simulink model "MCVT_Integration"  (R2020a)
settings: Solver=ode8  StartTime=0.0  StopTime=T_end  FixedStep=dt  RelTol=1e-3  EnableMultiTasking=on
values from neighbouring files (symbols this model uses):
  assumed_power_voltage = 1.25e2    (= 125)    [MCVT_Input_File.m]    (also in MCVT_Run_QuickCheck.m)
  battery_power_toggle = 1    [MCVT_Input_File.m]    (also in MCVT_Run_QuickCheck.m)
  battery_trickle_charge_param = 0.1    [MCVT_Input_File.m]    (also in MCVT_Run_QuickCheck.m)
  battery_trickle_leakage_param = 0.01    [MCVT_Input_File.m]    (also in MCVT_Run_QuickCheck.m)
  damage_case_id = 3    [MCVT_Input_File.m]    (also in MCVT_Run_QuickCheck.m = 1)
  dt = 1/fs    (= 0.001)    [MCVT_Run_QuickCheck.m]    (also in MCVT_Sim_Set.m)
  dt_2 = 1/fs_2    (= 0.001)    [MCVT_Run_QuickCheck.m]    (also in MCVT_Sim_Set.m)
  dt_3 = 1/fs_3    (= 0.001)    [MCVT_Run_QuickCheck.m]    (also in MCVT_Sim_Set.m)
  dt_5 = 1/fs_5    (= 0.005)    [MCVT_Run_QuickCheck.m]    (also in MCVT_Sim_Set.m)
  dt_8 = 1/fs_8    (= 0.05)    [MCVT_Run_QuickCheck.m]    (also in MCVT_Sim_Set.m)
  dt_9 = 1/fs_9    (= 0.001)    [MCVT_Run_QuickCheck.m]    (also in MCVT_Sim_Set.m)
  dust_met_duration = 3    [MCVT_Input_File.m]    (also in MCVT_Run_QuickCheck.m = 2)
  ECLSS_indoor_set_temperature = 298.15    [MCVT_Input_File.m]    (also in MCVT_Run_QuickCheck.m)
  energy_store_max_o = 150    [MCVT_Input_File.m]    (also in MCVT_Run_QuickCheck.m)
  energy_store_o = 120    [MCVT_Input_File.m]    (also in MCVT_Run_QuickCheck.m)
  fs = 1000    [MCVT_Run_QuickCheck.m]    (also in MCVT_Sim_Set.m)
  gain_charge_param = 1    [MCVT_Input_File.m]    (also in MCVT_Run_QuickCheck.m)
  ground_temperature = 373    [MCVT_Habitat_Design_Input_File.m]    (also in MCVT_Run_QuickCheck.m)
  I_max_charge = 800    [MCVT_Input_File.m]    (also in MCVT_Run_QuickCheck.m)
  I_max_discharge = 800    [MCVT_Input_File.m]    (also in MCVT_Run_QuickCheck.m)
  initial_pressure = 1e5    (= 100000)    [MCVT_Input_File.m]    (also in MCVT_Run_QuickCheck.m)
  initial_temperature = 295    [MCVT_Input_File.m]    (also in MCVT_Run_QuickCheck.m)
  interior_leak_rate = 0.00415    [MCVT_Input_File.m]    (also in MCVT_Run_QuickCheck.m)
  launch_dust_accum_start_time = 10    [MCVT_Input_File.m]    (also in MCVT_Run_QuickCheck.m = 2)
  launch_dust_accum_stop_time = 12    [MCVT_Input_File.m]    (also in MCVT_Run_QuickCheck.m = 3)
  meteorite_impact_time = 2    [MCVT_Input_File.m]    (also in MCVT_Run_QuickCheck.m = 1)
  nuclear_power_toggle = 1    [MCVT_Input_File.m]    (also in MCVT_Run_QuickCheck.m)
  print_interval = 30    [MCVT_Input_File.m]
  SOC_lower_thres = 0.5    [MCVT_Input_File.m]    (also in MCVT_Run_QuickCheck.m)
  SOC_upper_thres = 0.9    [MCVT_Input_File.m]    (also in MCVT_Run_QuickCheck.m)
  solar_power_toggle = 1    [MCVT_Input_File.m]    (also in MCVT_Run_QuickCheck.m)
  space_temperature = 4    [MCVT_Habitat_Design_Input_File.m]    (also in MCVT_Run_QuickCheck.m)
  Start_angle = 90    [MCVT_Input_File.m]    (also in MCVT_Run_QuickCheck.m)
undefined symbols (not defined in the file or in its folder): A_flow_restrictor, ACTIVITY_LOOKUP_TABLE, air, Aref, Bmap, Bmap2, Bmap3, comms, DiscIntNeverNeededParam, DmgSPL, dust_parameters, ECLSS_dust_damage_repair_time, ECLSS_heater_capacity, ECLSS_IPS_restrictor, ECLSS_IPS_setpointPressure, ECLSS_paint_damage_repair_time, ECLSS_radiatorLoop_discreteNumber, ECLSS_Vst, emptyActivity, expected_heat_add, FI, habitat, HIEM2SMM, HIEM2STM, HIEMDamageElements, impact_numbering, initial_SPLT_damage_index, input_air, input_Cond, input_CP, input_discretization, input_EV, input_Evap, input_radiatorLoop, K_ECLSS_dust_single, K_ECLSS_paint_single, K_Structure_damage_single, Kref, kW_to_W, meteoroidDustDep, mFRF, ndelays, nominalDustDep, noverlap, nuclear_toggle_gain, output_limits, panel_paint0, Ps0, reference_temperature, RepairTime_ECLSS_factor, Sensor_Location_SMM, SMM_T0, SMM_T1, SMM_T2, SMM_T3, SMM_T4, SMM_T4_0, SMM_T4_1, SMM_T4_2, solar_FDD_K ... (+73)
libraries: neural x350, simulink x39, xpcutilitieslib x7, xpcrtudplib x7, dspstat3 x1
(part 3 of 43)

== system MCVT_Integration/Sys 6 - Health Management and Agents/ Agent System/Agent Module ==
  Intervention_Pgm : Inport
  Action Scheduler : Subsystem
  Agent Memory : Subsystem
  Bus Creator : BusCreator  Inputs=3
  Bus Creator1 : BusCreator  Inputs=3
  MATLAB Function : MATLAB Function  TreatAsAtomicUnit=on
  Plant Model : Subsystem
  Terminator : Terminator
  Unit Delay : UnitDelay  InitialCondition=false  SampleTime=-1  HasFrameUpgradeWarning=on
  programInfo : Outport
  Agent_Memory_States : Outport  Port=2
  AgentPlantStates : Outport  Port=3
  AgentComms : Outport  Port=4
  wires:
  Action Scheduler/3(currActParams) -> Plant Model/2(actionParams)
  Action Scheduler/5(actSampleTime) -> Plant Model/4(actSampleTime)
  Unit Delay/1 -> Agent Memory/1(currAcvtDone)
  Intervention_Pgm/1 -> Agent Memory/2(Intervention_Pgm)
  Action Scheduler/2(currActID) -> MATLAB Function/1(actionID), Bus Creator1/2, Plant Model/1(actionID)  "actionID"
  Action Scheduler/1(currAcvtDone) -> Unit Delay/1
  Action Scheduler/4(currActDone) -> Plant Model/3(actionDone)
  Agent Memory/1(currPgm) -> Action Scheduler/1(CURR_TODO)  "CURR_TODO"
  Plant Model/2(Comms_SubSys_Combined) -> AgentComms/1
  Agent Memory/4(currAcvtID) -> Bus Creator/3, Bus Creator1/1  "currAcvtID"
  Plant Model/1(States) -> AgentPlantStates/1
  MATLAB Function/1(cActionID) -> Bus Creator1/3  "cActionID"
  Bus Creator/1 -> Agent_Memory_States/1
  Agent Memory/3(activityIDs) -> Bus Creator/2  "Activity_IDs"
  Bus Creator1/1 -> programInfo/1
  Agent Memory/2(filledMemSlots) -> Bus Creator/1  "Filled_Memory_Slots"
  Plant Model/3(action_done_flags) -> Terminator/1

== system MCVT_Integration/Sys 6 - Health Management and Agents/ Agent System/Interact_with_Susystems ==
  note: Agent Interactions with Electro-Mechanical Subsystems
      | The `Agent_Subsys_Comms` arre packaged as follows:
      | -------------------------------------------------------------------------
      | | Index | Relevant Electro-mechanical Susystem |
      | --------------------------------------------------------------------------
      | | 1 | Nuclear Power Generation |
      | | 2 | Solar Power Generation |
      | | 3 | Structure |
      | | 4 | ECLSS |
      | --------------------------------------------------------------------------
  Agent_SubSys_Comms : Inport
  AgentCommBus : BusCreator  Inputs=4
  Selector : Selector  NumberOfDimensions=2  InputPortWidth=3  IndexOptions=Select all,Index vector (dialog)  Indices=[1 3],1  OutputSizes=1,1
  Selector1 : Selector  NumberOfDimensions=2  InputPortWidth=3  IndexOptions=Select all,Index vector (dialog)  Indices=[1 3],2  OutputSizes=1,1
  Selector2 : Selector  NumberOfDimensions=2  InputPortWidth=3  IndexOptions=Select all,Index vector (dialog)  Indices=[1 3],3  OutputSizes=1,1
  Selector3 : Selector  NumberOfDimensions=2  InputPortWidth=3  IndexOptions=Select all,Index vector (dialog)  Indices=[1 3],4  OutputSizes=1,1
  Agent_Comms : Outport
  wires:
  AgentCommBus/1 -> Agent_Comms/1
  Agent_SubSys_Comms/1 -> Selector/1, Selector1/1, Selector3/1, Selector2/1
  Selector2/1 -> AgentCommBus/3  "Repair_Comms_Structure"
  Selector3/1 -> AgentCommBus/4  "Repair_Comms_ECLSS"
  Selector/1 -> AgentCommBus/1  "Repair_Comms_NPG"
  Selector1/1 -> AgentCommBus/2  "Repair_Comms_SPG"

== system MCVT_Integration/Sys 6 - Health Management and Agents/ Agent System/Viz ==
  AgentStates : Inport
  Agent_Comms : Inport  Port=2
  Agent Interaction : Scope  NumInputPorts=4
  Agent Memory : Scope  NumInputPorts=3
  Agent States : Scope  NumInputPorts=3
  Bus Selector : BusSelector  OutputSignals=Agent_Program_Info.currAcvtID,Agent_Program_Info.cActionID,Agent_Memory_States.Filled_Memory_Slots,Agent_Plant_States
  Bus Selector1 : BusSelector  OutputSignals=Repair_Comms_NPG,Repair_Comms_SPG,Repair_Comms_Structure,Repair_Comms_ECLSS
  Selector : Selector  InputPortWidth=3  IndexOptions=Index vector (dialog)  Indices=1  OutputSizes=1
  Selector1 : Selector  InputPortWidth=3  IndexOptions=Index vector (dialog)  Indices=2  OutputSizes=1
  Selector2 : Selector  InputPortWidth=3  IndexOptions=Index vector (dialog)  Indices=3  OutputSizes=1
  wires:
  AgentStates/1 -> Bus Selector/1
  Agent_Comms/1 -> Bus Selector1/1
  Bus Selector1/1 -> Agent Interaction/1  "<Repair_Comms_NPG>"
  Bus Selector1/2 -> Agent Interaction/2  "<Repair_Comms_SPG>"
  Bus Selector1/3 -> Agent Interaction/3  "<Repair_Comms_Structure>"
  Bus Selector1/4 -> Agent Interaction/4  "<Repair_Comms_ECLSS>"
  Bus Selector/1 -> Agent Memory/1  "<currAcvtID>"
  Bus Selector/2 -> Agent Memory/2  "<cActionID>"
  Bus Selector/3 -> Agent Memory/3  "<Filled_Memory_Slots>"
  Bus Selector/4 -> Selector2/1, Selector1/1, Selector/1  "<Agent_Plant_States>"
  Selector/1 -> Agent States/1  "soc"
  Selector1/1 -> Agent States/2  "mobilityHS"
  Selector2/1 -> Agent States/3  "position"

== system MCVT_Integration/Sys 6 - Health Management and Agents/Decision Maker/Simulink Communication Modules ==
  note: Sockets for FDD - ECLSS
  note: Sockets for FDD - Power Systems
  note: Socket for FDD - Structural System
  note: Simulated / Physics-based FDD Time Signatures at simulation frequency (fs = 1000 Hz)
  note: Simulink Communication APIs
  Sub6_Cyber_Inputs : Inport
  Agent_States : Inport  Port=2
  Agent/MCVT API from HMS : Subsystem
  Agent/MCVT API to HMS : Subsystem
  Bus Selector : BusSelector  OutputSignals=Sys2_out_DamageProbabilities.Sys2_Out_FDD_Structure_Damage_xe,Sys3_Out_PowerSystemDamageInformation.SolarPV_FDD_simu,Sys3_Out_PowerSystemDamageInformation.Nuclear_Dust_FDD,Sys5_Out_Damage_Info.FDD_ECLSS_Dust_xe,Sys5_Out_Damage_Info.FDD_ECLSS_Paint_xe
  Bus Selector1 : BusSelector  OutputSignals=Agent_Memory_States.currAcvtID
  Constant : Constant  Value=0.1
  Constant1 : Constant  Value=0.1*ones(4,1)
  Constant2 : Constant  Value=0
  ECLSS_Dust/MCVT API to HMS : Subsystem
  ECLSS_Paint/MCVT API to HMS : Subsystem
  NPG_Dust/MCVT API to HMS : Subsystem
  Solar_PV/MCVT API to HMS : Subsystem
  Structure_Dmg/MCVT API to HMS : Subsystem
  HMS_Suggested_Activity : Outport
  wires:
  Sub6_Cyber_Inputs/1 -> Bus Selector/1
  Bus Selector/1 -> [FDD_Str_Dmg_xe]  "<Sys2_Out_FDD_Structure_Damage_xe>"
  Bus Selector/2 -> [FDD_SPG_Dust_xe]  "<SolarPV_FDD_simu>"
  Bus Selector/3 -> [FDD_NPG_Dust]  "<Nuclear_Dust_FDD>"
  Bus Selector/4 -> [FDD_ECLSS_Dust_xe]  "<FDD_ECLSS_Dust_xe>"
  Bus Selector/5 -> [FDD_ECLSS_Paint_xe]  "<FDD_ECLSS_Paint_xe>"
  [FDD_ECLSS_Dust_xe] -> ECLSS_Dust/MCVT API to HMS/1(ECLSS_Dust_xe)
  [FDD_ECLSS_Paint_xe] -> ECLSS_Paint/MCVT API to HMS/1(ECLSS_Paint_xe)
  Agent_States/1 -> Bus Selector1/1
  Bus Selector1/1 -> [Agent_States]  "<currAcvtID>"
  [Agent_States] -> Agent/MCVT API to HMS/1(Agent_States)
  Agent/MCVT API from HMS/1(HMS_Suggested_Activity) -> HMS_Suggested_Activity/1
  Constant/1 -> Structure_Dmg/MCVT API to HMS/1(Structure_FDD_Damage_xe)
  Constant1/1 -> Solar_PV/MCVT API to HMS/1(FDD_SPG_Dust_xe)
  Constant2/1 -> NPG_Dust/MCVT API to HMS/1(FDD_NPG_Dust)

== system MCVT_Integration/Sys 6 - Health Management and Agents/Decision Maker/Viz ==
  Sub6_Cyber_Inputs : Inport
  Sys6_Phy_Inputs : Inport  Port=2
  Bus Selector : BusSelector  OutputSignals=Sys2_out_DamageProbabilities.Sys2_Out_FDD_Structure_Damage_xe,Sys3_Out_PowerSystemDamageInformation.SolarPV_FDD_simu,Sys3_Out_PowerSystemDamageInformation.Nuclear_Dust_FDD,Sys5_Out_Damage_Info.FDD_ECLSS_Dust_xe,Sys5_Out_Damage_Info.FDD_ECLSS_Paint_xe,Sys2_out_DamageProbabilities.Sys2_Out_PanelDamageProbabilities
  Bus Selector1 : BusSelector  OutputSignals=Sys2_Total_Damage_to_Sys6,Sys3_Out_Physical_Inputs_Agents.Sub3_solarPV_DRout,Sys3_Out_Physical_Inputs_Agents.Sub3_Nuclear_Out_Accumulated_Dust_mg_cm2
  FDD Outputs : Scope  NumInputPorts=6
  Physical States : Scope  NumInputPorts=3
  wires:
  Sub6_Cyber_Inputs/1 -> Bus Selector/1
  Bus Selector/1 -> FDD Outputs/1  "<Sys2_Out_FDD_Structure_Damage_xe>"
  Bus Selector/2 -> FDD Outputs/2  "<SolarPV_FDD_simu>"
  Bus Selector/3 -> FDD Outputs/3  "<Nuclear_Dust_FDD>"
  Bus Selector/4 -> FDD Outputs/4  "<FDD_ECLSS_Dust_xe>"
  Bus Selector/5 -> FDD Outputs/5  "<FDD_ECLSS_Paint_xe>"
  Sys6_Phy_Inputs/1 -> Bus Selector1/1
  Bus Selector1/1 -> Physical States/1  "<Sys2_Total_Damage_to_Sys6>"
  Bus Selector1/2 -> Physical States/2  "<Sub3_solarPV_DRout>"
  Bus Selector1/3 -> Physical States/3  "<Sub3_Nuclear_Out_Accumulated_Dust_mg_cm2>"
  Bus Selector/6 -> FDD Outputs/6  "<Sys2_Out_PanelDamageProbabilities>"

== system MCVT_Integration/Sys 6 - Health Management and Agents/Decision Maker/module3 ==
  Sub6_Cyber_Inputs : Inport
  Bus Selector : BusSelector  OutputSignals=Sys3_Out_PowerSystemDamageInformation.Nuclear_Dust_FDD,Sys3_Out_PowerSystemDamageInformation.SolarPV_FDD,Sys2_out_DamageProbabilities.Sys2_Out_PanelDamageProbabilities
  Mux : Mux  Inputs=3
  test_inputs : Outport
  wires:
  Sub6_Cyber_Inputs/1 -> Bus Selector/1
  Bus Selector/1 -> Mux/1  "<Nuclear_Dust_FDD>"
  Bus Selector/2 -> Mux/2  "<SolarPV_FDD>"
  Bus Selector/3 -> Mux/3  "<Sys2_Out_PanelDamageProbabilities>"
  Mux/1 -> test_inputs/1

== system MCVT_Integration/Sys2 - Structural System/Sys2_Structural_System/3D Structural Protective Layer-Thermal (SPL-T) ==
  Sys2.4_STM2SPL_MemoryBock : Inport
  Sys2.4_In_Solar_Irradiation_(from_Sys9) : Inport  Port=2
  Sys2.4_In_Damage_SPL : Inport  Port=3
  Sys2.4_In_Meteorite_Impact_Location : Inport  Port=4
  Clock : Clock
  Fir_SPL : ToWorkspace  VariableName=Fir_SPL  SampleTime=-1
  Sec_SPL : ToWorkspace  VariableName=Sec_SPL  SampleTime=-1
  Start_angle : Constant  Value=Start_angle
  Structural Protective Layer-Thermal (SPL-T) : MATLABSystem  System=SPLT  emis_reg=regolith.emissivity  abs_reg=regolith.absorptivity  sigma=sb_sigma  rho_reg=regolith.density  k_reg=regolith.thermal_conductivity  c_reg=regolith.specific_heat  k_con=concrete.thermal_conductivity  Tspace=space_temperature  R=habitat.inner_radius+habitat.dome_thickness  d=habitat.spl_thickness  d_STM=habitat.dome_thickness  Trepair=repair_temperature  Tfoundation=foundation_temperature  SimulateUsing=Code generation
      mask Type: SPLT
      mask Display:
      | disp('SPLT');
      | port_label('input',1,'STM2SPL1');
      | port_label('input',2,'Sb_tot');
      | port_label('input',3,'t');
      | port_label('input',4,'dt_2');
      | port_label('input',5,'Dam_SPL');
      | port_label('input',6,'ImpLoc');
      | port_label('input',7,'Start_angle');
      | port_label('input',8,'T_SPL_initialize');
      | port_label('output',1,'SPL2STM');
      | port_label('output',2,'Index_Damage');
      | port_label('output',3,'T_SPL_Ext');
      | port_label('output',4,'T_Fir');
      | port_label('output',5,'T_Sec');
      | port_label('output',6,'T_Thi');
      | port_label('output',7,'SPL_Sec');
      | port_label('output',8,'Fir_SPL');
      | port_label('output',9,'Sec_SPL');
      | port_label('output',10,'Thi_SPL');
  T_Fir : Scope  Commented=on
  T_SPL_initialize : Constant  Value=T_SPL_initialize  VectorParams1D=off
  T_Sec : Scope  Commented=on
  T_Thi : Scope  Commented=on
  Thi_SPL : ToWorkspace  VariableName=Thi_SPL  SampleTime=-1
  Time step : Constant  Value=dt_2
  Sys2.4_SPL2STM_MemoryBlock : Outport
  Sys2.4_DamageElementNumber_SPLT_to_STM : Outport  Port=2
  Sys2.4_out_ExteriorWallTemp : Outport  Port=3
  wires:
  Sys2.4_STM2SPL_MemoryBock/1 -> Structural Protective Layer-Thermal (SPL-T)/1
  Structural Protective Layer-Thermal (SPL-T)/3 -> Sys2.4_out_ExteriorWallTemp/1
  Structural Protective Layer-Thermal (SPL-T)/6 -> T_Thi/1
  Structural Protective Layer-Thermal (SPL-T)/8 -> Fir_SPL/1
  Clock/1 -> Structural Protective Layer-Thermal (SPL-T)/3
  Start_angle/1 -> Structural Protective Layer-Thermal (SPL-T)/7
  Structural Protective Layer-Thermal (SPL-T)/10 -> Thi_SPL/1
  Sys2.4_In_Solar_Irradiation_(from_Sys9)/1 -> Structural Protective Layer-Thermal (SPL-T)/2
  Structural Protective Layer-Thermal (SPL-T)/5 -> T_Sec/1
  Structural Protective Layer-Thermal (SPL-T)/4 -> T_Fir/1
  T_SPL_initialize/1 -> Structural Protective Layer-Thermal (SPL-T)/8
  Structural Protective Layer-Thermal (SPL-T)/9 -> Sec_SPL/1
  Time step/1 -> Structural Protective Layer-Thermal (SPL-T)/4
  Structural Protective Layer-Thermal (SPL-T)/1 -> Sys2.4_SPL2STM_MemoryBlock/1
  Sys2.4_In_Meteorite_Impact_Location/1 -> Structural Protective Layer-Thermal (SPL-T)/6
  Sys2.4_In_Damage_SPL/1 -> Structural Protective Layer-Thermal (SPL-T)/5
  Structural Protective Layer-Thermal (SPL-T)/2 -> Sys2.4_DamageElementNumber_SPLT_to_STM/1

== system MCVT_Integration/Sys2 - Structural System/Sys2_Structural_System/FDD adjustment ==
  x : Inport
  Clock2 : Clock
  FDDS : MATLAB Function  TreatAsAtomicUnit=on
  FDDSr : MATLAB Function  TreatAsAtomicUnit=on
  Scope1 : Scope
  Tapped Delay : simulink/Discrete/Tapped Delay  vinit=0.0  samptime=-1  NumDelays=10/dt_2  DelayOrder=Oldest  includeCurrent=off
  y : Outport
  wires:
  x/1 -> FDDS/1(x)
  Tapped Delay/1 -> FDDSr/1(x)
  Clock2/1 -> FDDS/2(t)
  FDDS/1(Y) -> Tapped Delay/1
  FDDSr/1(Y) -> Scope1/1, y/1

== system MCVT_Integration/Sys2 - Structural System/Sys2_Structural_System/Fault Detection and Diagnosis (FDD) 2.3 ==
  Sys2.3_In_Excitation (White Noise) : Inport
  Sys2.3_In_Acceleration Response : Inport  Port=2
  Sys2.3_In_Accelerometer Power Supply (kW) : Inport  Port=3
  Accelerometer : Subsystem
  Accelerometer Power Consumption : MATLAB Function  TreatAsAtomicUnit=on
  Data Anomaly Detection : Subsystem  TreatAsAtomicUnit=on
  "Excitation Delay 1 " : simulink/Discrete/Tapped Delay  vinit=0.0  samptime=-1  NumDelays=ndelays  DelayOrder=Oldest  includeCurrent=off
  FRF from Accelerometer 1 : Subsystem  TreatAsAtomicUnit=on
  FRF from Accelerometer 2 : Subsystem  TreatAsAtomicUnit=on
  FRF from Accelerometer 3 : Subsystem  TreatAsAtomicUnit=on
  Pattern Recognition Neural Network : Subsystem  TreatAsAtomicUnit=on
  "Pulse Generator " : DiscretePulseGenerator  Period=trigger  PhaseDelay=trigger  SampleTime=Time_step
  Pulse Generator 1 : DiscretePulseGenerator  Period=trigger  PhaseDelay=trigger+1  SampleTime=Time_step
  Pulse Generator 2 : DiscretePulseGenerator  Period=trigger  PhaseDelay=trigger+2  SampleTime=Time_step
  Pulse Generator 3 : DiscretePulseGenerator  Period=trigger  PhaseDelay=trigger+2  SampleTime=Time_step
  Pulse Generator 4 : DiscretePulseGenerator  Period=ndelays  PhaseDelay=ndelays  SampleTime=Time_step
  Sys2.3_Out_Probabilities : Outport
  Sys2.3_Out_Accelerometer Power Consumption (kW) : Outport  Port=2
  wires:
  Accelerometer/1(Out2) -> Data Anomaly Detection/1("Measured Acceleration "), FRF from Accelerometer 2/2(Acceleration Response), FRF from Accelerometer 1/2(Acceleration Response), FRF from Accelerometer 3/2(Acceleration Response)
  Sys2.3_In_Acceleration Response/1 -> Accelerometer/1(Actural Acceleration)
  "Pulse Generator "/1 -> FRF from Accelerometer 1/Trigger
  "Excitation Delay 1 "/1 -> FRF from Accelerometer 3/1(Excitation (White Noise)), FRF from Accelerometer 2/1(Excitation (White Noise)), FRF from Accelerometer 1/1(Excitation (White Noise))
  Sys2.3_In_Excitation (White Noise)/1 -> "Excitation Delay 1 "/1
  Pulse Generator 1/1 -> FRF from Accelerometer 2/Trigger
  Pulse Generator 2/1 -> FRF from Accelerometer 3/Trigger
  Pattern Recognition Neural Network/1(Damage Probabilities) -> Sys2.3_Out_Probabilities/1
  Pulse Generator 3/1 -> Pattern Recognition Neural Network/Trigger
  FRF from Accelerometer 3/1(Out1) -> Pattern Recognition Neural Network/3(FRF 3)
  FRF from Accelerometer 1/1(Out1) -> Pattern Recognition Neural Network/1(FRF 1)
  FRF from Accelerometer 2/1(Out1) -> Pattern Recognition Neural Network/2(FRF 2)
  Pulse Generator 4/1 -> Data Anomaly Detection/Trigger
  Sys2.3_In_Accelerometer Power Supply (kW)/1 -> Accelerometer Power Consumption/1(p), Accelerometer/2(Accelerometer Power Supply)
  Accelerometer Power Consumption/1(y) -> Sys2.3_Out_Accelerometer Power Consumption (kW)/1

== system MCVT_Integration/Sys2 - Structural System/Sys2_Structural_System/Fault Detection and Diagnosis (FDD) with Binary Classifer ==
  Sys2.3_In_Excitation (White Noise) : Inport
  Sys2.3_In_Acceleration Response : Inport  Port=2
  Sys2.3_In_Accelerometer Power Supply (kW) : Inport  Port=3
  Sys2.3_In_Repair_Status : Inport  Port=4
  Accelerometer : Subsystem
  Accelerometer Power Consumption : MATLAB Function  TreatAsAtomicUnit=on
  Clock : Clock
  Clock1 : Clock
  Constant : Constant  Value=meteorite_impact_time
  Damage Probabilities {1..5} (5 alike) : Display  Commented=on
  Data Anomaly Detection Results : Display  Commented=on
  Data Anomaly Detection : Subsystem  TreatAsAtomicUnit=on
  "Excitation Delay 1 " : simulink/Discrete/Tapped Delay  vinit=0.0  samptime=-1  NumDelays=ndelays  DelayOrder=Oldest  includeCurrent=off
  FRF from Accelerometer 1 : Subsystem  TreatAsAtomicUnit=on
  FRF from Accelerometer 2 : Subsystem  TreatAsAtomicUnit=on
  FRF from Accelerometer 3 : Subsystem  TreatAsAtomicUnit=on
  Find repair start time : MATLAB Function  TreatAsAtomicUnit=on
  Mux : Mux  Inputs=5
  Pattern Recognition Neural Network : Subsystem  TreatAsAtomicUnit=on
  Post-processing Block : MATLAB Function  TreatAsAtomicUnit=on
  "Pulse Generator " : DiscretePulseGenerator  Period=trigger  PhaseDelay=trigger  SampleTime=Time_step
  Pulse Generator 1 : DiscretePulseGenerator  Period=trigger  PhaseDelay=trigger+1  SampleTime=Time_step
  Pulse Generator 2 : DiscretePulseGenerator  Period=trigger  PhaseDelay=trigger+2  SampleTime=Time_step
  Pulse Generator 3 : DiscretePulseGenerator  Period=trigger  PhaseDelay=trigger+2  SampleTime=Time_step
  Pulse Generator 4 : DiscretePulseGenerator  Period=ndelays  PhaseDelay=ndelays  SampleTime=Time_step
  Scope : Scope
  Subsystem : Subsystem
  Sys2.3_Out_Accelerometer Power Consumption : Outport
  Sys2.3_Out_Damage_Detection_Probabilities : Outport  Port=2
  Sys2.3_Out_Impact_location_Probabilities : Outport  Port=3
  wires:
  Accelerometer/1(Acceleration_delay) -> Data Anomaly Detection/1("Measured Acceleration "), FRF from Accelerometer 2/2(Acceleration Response), FRF from Accelerometer 1/2(Acceleration Response), FRF from Accelerometer 3/2(Acceleration Response)
  Sys2.3_In_Acceleration Response/1 -> Accelerometer/1(Actural Acceleration)
  "Pulse Generator "/1 -> FRF from Accelerometer 1/Trigger
  "Excitation Delay 1 "/1 -> FRF from Accelerometer 3/1(Excitation (White Noise)), FRF from Accelerometer 2/1(Excitation (White Noise)), FRF from Accelerometer 1/1(Excitation (White Noise))
  Sys2.3_In_Excitation (White Noise)/1 -> "Excitation Delay 1 "/1
  Pulse Generator 1/1 -> FRF from Accelerometer 2/Trigger
  Pulse Generator 2/1 -> FRF from Accelerometer 3/Trigger
  Pattern Recognition Neural Network/1(Damage Probabilities Segment 1) -> Mux/1, Damage Probabilities 1/1
  Pulse Generator 3/1 -> Pattern Recognition Neural Network/Trigger
  FRF from Accelerometer 3/1(Out1) -> Pattern Recognition Neural Network/3(FRF 3)
  FRF from Accelerometer 1/1(Out1) -> Pattern Recognition Neural Network/1(FRF 1)
  FRF from Accelerometer 2/1(Out1) -> Pattern Recognition Neural Network/2(FRF 2)
  Pulse Generator 4/1 -> Data Anomaly Detection/Trigger
  Data Anomaly Detection/1(indicator) -> Data Anomaly Detection Results/1
  Sys2.3_In_Accelerometer Power Supply (kW)/1 -> Accelerometer Power Consumption/1(p), Accelerometer/2(Accelerometer Power Supply)
  Accelerometer Power Consumption/1(y) -> Sys2.3_Out_Accelerometer Power Consumption/1
  Pattern Recognition Neural Network/2(Damage Probabilities Segment 2) -> Mux/2, Damage Probabilities 2/1
  Pattern Recognition Neural Network/3(Damage Probabilities Segment 3) -> Damage Probabilities 3/1, Mux/3
  Pattern Recognition Neural Network/4(Damage Probabilities Segment 4) -> Damage Probabilities 4/1, Mux/4
  Pattern Recognition Neural Network/5(Damage Probabilities Segment 5) -> Damage Probabilities 5/1, Mux/5
  Subsystem/1(Impact Location) -> Sys2.3_Out_Impact_location_Probabilities/1
  Accelerometer/2(Acceleration_origin) -> Subsystem/1(Acceleration)
  Sys2.3_In_Repair_Status/1 -> Find repair start time/1(u), Subsystem/2(Repair)
  Post-processing Block/1(y) -> Scope/1, Sys2.3_Out_Damage_Detection_Probabilities/1
  Clock/1 -> Post-processing Block/2(t)
  Mux/1 -> Post-processing Block/1(u)
  Constant/1 -> Post-processing Block/4(impact_begin)
  Clock1/1 -> Find repair start time/2(t)
  Find repair start time/1(y) -> Post-processing Block/3(repair_begin)

== system MCVT_Integration/Sys2 - Structural System/Sys2_Structural_System/Sim FDD Damage Detection ==
  Sys2_Total_Damage : Inport
  EnableResetFDD_Structure : Inport  Port=2
  MATLAB Function : MATLAB Function  TreatAsAtomicUnit=on
  MATLAB System : MATLABSystem  System=Structure_simu_FDD_damage  timestep=[dt_2]  Ksingle=[K_Structure_damage_single]  noise=1e-2  num=1  SimulateUsing=Code generation
      mask Type: Structure_simu_FDD_damage
      mask Display:
      | disp('Structure_simu_FDD_damage');
      | port_label('input',1,'xtrue');
      | port_label('input',2,'EnableReset');
      | port_label('output',1,'xe');
  FDD_Structure_Damage_xe : Outport
  wires:
  Sys2_Total_Damage/1 -> MATLAB Function/1(u)
  EnableResetFDD_Structure/1 -> MATLAB System/2
  MATLAB System/1 -> FDD_Structure_Damage_xe/1
  MATLAB Function/1(xtrue) -> MATLAB System/1

== system MCVT_Integration/Sys2 - Structural System/Sys2_Structural_System/Structural Mechanical 2.1 ==
  note: SMM Solver
  note: Force Calculation
  note: Domain Selection
  Sys2.1_In_Temp_Profile : Inport
  Sys2.1_In_Internal_Pressure : Inport  Port=2
  Sys2.1_In_Impact_Force : Inport  Port=3
  Sys_2.1_In_Impact_Location : Inport  Port=4
  Sys2.1_In_Moonquake_Acceleration : Inport  Port=5
  Sys2.1_In_White Noise : Inport  Port=6
  Sys2.1_In_Repair_Status : Inport  Port=7
  Cat Acceleration : MATLAB Function  TreatAsAtomicUnit=on
  Clock1 : Clock
  Clock2 : Clock
  Domain Selection : MATLAB Function  TreatAsAtomicUnit=on
  Force Calculation : MATLAB Function  TreatAsAtomicUnit=on
  MATLAB Function : MATLAB Function  TreatAsAtomicUnit=on
  Scope : Scope  Commented=on
  Scope1 : Scope  Commented=on
  Solver : MATLAB Function  TreatAsAtomicUnit=on
  Unit Delay : UnitDelay  InitialCondition=zeros(SMM_T1.neq,1)  SampleTime=-1  HasFrameUpgradeWarning=on
  Unit Delay1 : UnitDelay  InitialCondition=zeros(max(SMM_T0.Type(3,:)),SMM_T1.nel)  SampleTime=-1  HasFrameUpgradeWarning=on
  Unit Delay10 : UnitDelay  InitialCondition=1  SampleTime=-1  HasFrameUpgradeWarning=on
  Unit Delay11 : UnitDelay  InitialCondition=1  SampleTime=-1  HasFrameUpgradeWarning=on
  Unit Delay2 : UnitDelay  InitialCondition=SMM_T4.Kbar_M  SampleTime=-1  HasFrameUpgradeWarning=on
  Unit Delay3 : UnitDelay  InitialCondition=zeros(SMM_T3.L,1)  SampleTime=-1  HasFrameUpgradeWarning=on
  Unit Delay4 : UnitDelay  InitialCondition=zeros(SMM_T3.L,1)  SampleTime=-1  HasFrameUpgradeWarning=on
  Unit Delay5 : UnitDelay  InitialCondition=zeros(SMM_T3.L,1)  SampleTime=-1  HasFrameUpgradeWarning=on
  Unit Delay6 : UnitDelay  InitialCondition=zeros(SMM_T1.neq,1)  SampleTime=-1  HasFrameUpgradeWarning=on
  Unit Delay7 : UnitDelay  InitialCondition=zeros(max(SMM_T0.Type(2,:))*3,SMM_T1.nel)  SampleTime=-1  HasFrameUpgradeWarning=on
  Unit Delay8 : UnitDelay  InitialCondition=1  SampleTime=-1  HasFrameUpgradeWarning=on
  Unit Delay9 : UnitDelay  InitialCondition=zeros(SMM_T2.TGP,1)  SampleTime=-1  HasFrameUpgradeWarning=on
  Sys2.1_Out_Damage_Locations : Outport
  Sys2.1_Out_Acceleration : Outport  Port=2
  Sys2.1_Out_Total_Damage : Outport  Port=3
  wires:
  Solver/7(Damage_Status_O) -> Unit Delay9/1, Sys2.1_Out_Damage_Locations/1
  Force Calculation/5(eTO) -> Unit Delay8/1
  Unit Delay3/1 -> Solver/2(Acc0)
  Unit Delay6/1 -> Force Calculation/1(fT)
  Solver/5(D_EqO) -> Unit Delay1/1
  Force Calculation/2(In_Acc) -> Solver/7(In_Acc)
  Sys2.1_In_Moonquake_Acceleration/1 -> Force Calculation/7(MQ_Acc)
  Sys2.1_In_Impact_Force/1 -> Force Calculation/9(Imp_F), Solver/12(Im_F)
  Solver/4(KbarO) -> Unit Delay2/1
  Unit Delay4/1 -> Solver/3(Vel0)
  Force Calculation/4(fT_eO) -> Unit Delay7/1
  Sys2.1_In_Repair_Status/1 -> Solver/9(Repair_Status)
  Sys2.1_In_White Noise/1 -> Force Calculation/8(WN_Acc)
  Unit Delay8/1 -> Force Calculation/3(eT)
  Sys2.1_In_Internal_Pressure/1 -> Force Calculation/6(PIn)
  Unit Delay9/1 -> Solver/10(Damage_Status)
  Clock2/1 -> Domain Selection/4(t)
  Unit Delay11/1 -> Domain Selection/2(T_NodeI)
  Solver/1(Disp1) -> Unit Delay5/1
  Cat Acceleration/1(Acc_Sen) -> Sys2.1_Out_Acceleration/1
  Unit Delay7/1 -> Force Calculation/2(fT_e)
  Unit Delay/1 -> Solver/8(Fadd)
  Solver/3(Acc1) -> MATLAB Function/1(u), Cat Acceleration/1(Acc), Unit Delay3/1
  Unit Delay5/1 -> Solver/1(Disp0)
  Force Calculation/3(fTO) -> Unit Delay6/1
  Sys2.1_In_Temp_Profile/1 -> Force Calculation/4(TIn)
  Unit Delay1/1 -> Solver/5(D_Eq)
  Domain Selection/1(Dom) -> Force Calculation/5(Dom), Solver/11(Dom), Cat Acceleration/2(Dom), Unit Delay10/1
  Solver/6(FaddO) -> Unit Delay/1
  Unit Delay2/1 -> Solver/4(Kbar)
  Solver/2(Vel1) -> Unit Delay4/1
  Force Calculation/1(Ext_F) -> Solver/6(Ext_F)
  Unit Delay10/1 -> Domain Selection/3(DomI)
  Sys_2.1_In_Impact_Location/1 -> Domain Selection/1(ILxyz)
  Domain Selection/2(T_Node) -> Unit Delay11/1, Force Calculation/10(T_Node)
  Clock1/1 -> Solver/13(t)
  Solver/8(Total_Damage) -> Scope/1, Sys2.1_Out_Total_Damage/1
  MATLAB Function/1(y) -> Scope1/1

== system MCVT_Integration/Sys2 - Structural System/Sys2_Structural_System/Structural Thermal Model 2.2 ==
  note: STM Temperature Sensors
  Sys2.2_In_Interior_Surface_Temperature_(from_Sys8) : Inport  PortDimensions=60
  Sys2.2_SPL2STM_Mem : Inport  Port=2
  Sys2.2_Damage_Index_SPLT : Inport  Port=3
  Sys2.2_In_Solar_Irradiation_(from_Sys9) : Inport  Port=4
  Sys2.2_In_SensorPowerSupply : Inport  Port=5
  Sys2.2_In_Damage_SPL : Inport  Port=6
  Sys2.2_In_HabitatWallDamage : Inport  Port=7  PortDimensions=[324 1]
  "3D Structural Thermal Model " : MATLABSystem  System=STM  emis_reg=regolith.emissivity  abs_reg=regolith.absorptivity  sigma=sb_sigma  rho_reg=regolith.density  k_reg=regolith.thermal_conductivity  c_reg=regolith.specific_heat  Tspace=space_temperature  initial_temperature=initial_temperature  emis_con=concrete.emissivity  abs_con=concrete.absorptivity  rho_con=concrete.density  k_con=concrete.thermal_conductivity  c_con=concrete.specific_heat  h=air.natural_convective_coefficient  R=habitat.inner_radius  d=habitat.dome_thickness  d_SPL=habitat.spl_thickness  Trepair=repair_temperature  Tfoundation=foundation_temperature  SimulateUsing=Code generation
      mask Type: STM
      mask Display:
      | disp('STM');
      | port_label('input',1,'SPL2STM1');
      | port_label('input',2,'TempHIEM2ST');
      | port_label('input',3,'Dam_SMM');
      | port_label('input',4,'Dam_SPL');
      | port_label('input',5,'Sb_tot');
      | port_label('input',6,'Index_Damage');
      | port_label('input',7,'t');
      | port_label('input',8,'dt_2');
      | port_label('input',9,'Start_angle');
      | port_label('input',10,'T_STM_initialize');
      | port_label('input',11,'Num_Dam_Prev');
      | port_label('output',1,'STM2SPL');
      | port_label('output',2,'STM2HIEM');
      | port_label('output',3,'STM2SMM');
      | port_label('output',4,'T_Fir1');
      | port_label('output',5,'T_Sec2');
      | port_label('output',6,'T_Thi3');
      | port_label('output',7,'STM_Sec');
      | port_label('output',8,'Fir_STM');
      | port_label('output',9,'Sec_STM');
      | port_label('output',10,'Thi_STM');
      | port_label('output',11,'Num_Dam');
  Clock3 : Clock
  Fir_STM : ToWorkspace  VariableName=Fir_STM  SampleTime=-1
  Num_Dam_MemBlock : Memory
  STM Temperature Sensors : Subsystem
  STM2SMM : Scope  Commented=on
  Sec_STM : ToWorkspace  VariableName=Sec_STM  SampleTime=-1
  Start_angle : Constant  Value=Start_angle
  T_Fir1 : Scope  Commented=on
  T_STM_initialize : Constant  Value=T_STM_initialize  VectorParams1D=off
  T_Sec1 : Scope  Commented=on
  T_Thi1 : Scope  Commented=on
  Temp2T6 : Scope  Commented=on
  Thi_STM : ToWorkspace  VariableName=Thi_STM  SampleTime=-1
  Time step : Constant  Value=dt_2
  Sys2.2_out_TotalPowerConsumption (kW) : Outport
  Sys2.2_STM2SPL_Mem : Outport  Port=2
  Sys2.2_out_InteriorWallSurfTemp_Sensors : Outport  Port=3
  Sys2.2_Power_Consumption_by_Sensor (kW) : Outport  Port=4
  Sys2.2_out_InteriorWallSurfaceTemp_(to_Sys8) : Outport  Port=5
  Sys2.2_out_WallTempDistribution_toSMM (To Sys 2.1) : Outport  Port=6
  wires:
  Sys2.2_In_Interior_Surface_Temperature_(from_Sys8)/1 -> "3D Structural Thermal Model "/2
  "3D Structural Thermal Model "/2 -> STM Temperature Sensors/1(Temperature (K)), Temp2T6/1, Sys2.2_out_InteriorWallSurfaceTemp_(to_Sys8)/1
  STM Temperature Sensors/3(Total_Power_Consumption_STM (kW)) -> Sys2.2_out_TotalPowerConsumption (kW)/1
  Num_Dam_MemBlock/1 -> "3D Structural Thermal Model "/11
  "3D Structural Thermal Model "/11 -> Num_Dam_MemBlock/1
  "3D Structural Thermal Model "/10 -> Thi_STM/1
  "3D Structural Thermal Model "/9 -> Sec_STM/1
  "3D Structural Thermal Model "/4 -> T_Fir1/1
  "3D Structural Thermal Model "/8 -> Fir_STM/1
  Sys2.2_In_Solar_Irradiation_(from_Sys9)/1 -> "3D Structural Thermal Model "/5
  "3D Structural Thermal Model "/5 -> T_Sec1/1
  "3D Structural Thermal Model "/3 -> STM2SMM/1, Sys2.2_out_WallTempDistribution_toSMM (To Sys 2.1)/1
  "3D Structural Thermal Model "/6 -> T_Thi1/1
  STM Temperature Sensors/1(Measured_Temperature (K)) -> Sys2.2_out_InteriorWallSurfTemp_Sensors/1
  "3D Structural Thermal Model "/1 -> Sys2.2_STM2SPL_Mem/1
  Sys2.2_In_Damage_SPL/1 -> "3D Structural Thermal Model "/4
  STM Temperature Sensors/2(Power_Consumption_by_Sensor (kW)) -> Sys2.2_Power_Consumption_by_Sensor (kW)/1
  Sys2.2_Damage_Index_SPLT/1 -> "3D Structural Thermal Model "/6
  Sys2.2_SPL2STM_Mem/1 -> "3D Structural Thermal Model "/1
  Sys2.2_In_SensorPowerSupply/1 -> STM Temperature Sensors/2(Power_Input (kW))
  Time step/1 -> "3D Structural Thermal Model "/8
  Start_angle/1 -> "3D Structural Thermal Model "/9
  T_STM_initialize/1 -> "3D Structural Thermal Model "/10
  Clock3/1 -> "3D Structural Thermal Model "/7
  Sys2.2_In_HabitatWallDamage/1 -> "3D Structural Thermal Model "/3

== system MCVT_Integration/Sys3 - Power System/Power Generation Subsystem1/Nuclear Power Generation ==
  Sub3_In_Dust_Accumulation (mg/cm^2) : Inport
  Sub3_In_Intervention : Inport  Port=2
  Disturbances1 : Subsystem
  SP-100 Power Generation : Subsystem
  Sub3_Out_Power_Generated (kW) : Outport
  Sub3_Out_Nuclear_Damage_Information : Outport  Port=2
  Sub3_Out_Heat_Rejection : Outport  Port=3
  Sub3_Nuclear_Out_Accumulated_Dust (mg/cm^2) : Outport  Port=4
  wires:
  Disturbances1/1(Sub3_Internal_Disturbance) -> [internal_dist]
  Sub3_In_Intervention/1 -> [intervention2]
  SP-100 Power Generation/1(Sub3_Out_Physical_Interdependency) -> Sub3_Out_Power_Generated (kW)/1
  SP-100 Power Generation/3(Heat Rejection Fraction of Nominal) -> Sub3_Out_Heat_Rejection/1
  SP-100 Power Generation/2(Sub3_Out_Cyber_Interdependency) -> Sub3_Out_Nuclear_Damage_Information/1
  [dust_accum2] -> SP-100 Power Generation/2(Sub3_In_Dust_Accumulation (mg/cm^2))
  [internal_dist] -> SP-100 Power Generation/1(Sub3_In_Internal_Disturbance)
  Sub3_In_Dust_Accumulation (mg/cm^2)/1 -> [dust_accum2]
  [dust_accum2] -> Sub3_Nuclear_Out_Accumulated_Dust (mg/cm^2)/1
  [intervention2] -> SP-100 Power Generation/3(Sub3_In_Intervention)

== system MCVT_Integration/Sys3 - Power System/Power Generation Subsystem1/Power Distribution and Storage System ==
  Sub3_In_Power_Consumed_by_Sub2 (kW) : Inport
  Sub3_In_Power_Consumed_by_Sub5 (kW) : Inport  Port=2
  Sub3_In_Power_Consumed_by_Sub8 (kW) : Inport  Port=3
  solar_power (kW) : Inport  Port=4
  nuclear_power (kW) : Inport  Port=5
  Demux : Demux  Outputs=3
  Power Delivered to each EPCE : Subsystem
  Product : Product
  Subsystem : Subsystem
  Subsystem2 : Subsystem
  Sum : Sum  Inputs=|+++
  Terminator2 : Terminator
  Terminator3 : Terminator
  sub3_voltage : Constant  Value=assumed_power_voltage
  Sub3_Out_Power_Supply_Sub2 (kW) : Outport
  Sub3_Out_Power_Supply_Sub5 (kW) : Outport  Port=2
  Sub3_Out_Power_Supply_Sub8 (kW) : Outport  Port=3
  Sub3_Out_Current_Power_Stored (kWh) : Outport  Port=4
  wires:
  [pow_consump] -> Power Delivered to each EPCE/2(Sub3_In_Power_Consumption (kW))
  Demux/1 -> Sub3_Out_Power_Supply_Sub2 (kW)/1  "p_consumed_Sub2"
  Demux/2 -> Sub3_Out_Power_Supply_Sub5 (kW)/1  "p_consumed_Sub5"
  Demux/3 -> Sub3_Out_Power_Supply_Sub8 (kW)/1  "p_consumed_Sub8"
  Power Delivered to each EPCE/1(p_consumed (kW)) -> Demux/1  "p_consumed"
  [V] -> Subsystem/6(Voltage (V))
  [sub2] -> Subsystem/1(structure_power (kW))
  [sub5] -> Subsystem/2(ECLSS_power (kW))
  [sub8] -> Subsystem/3(interior_power (kW))
  [s_pow] -> Subsystem/4(P_out_solar (kW))
  [n_pow] -> Subsystem/5(P_out_nuclear (kW))
  [battery] -> Sub3_Out_Current_Power_Stored (kWh)/1
  [pow_avail] -> Power Delivered to each EPCE/1(total_power_avail (kW))  "total_power_avail"
  Subsystem/1(Solar Power (kW)) -> Sum/1  "sol_pow_gained"
  Subsystem/2(Nuclear Power (kW)) -> Sum/2  "nuc_pow_gained"
  Subsystem/4(time_discharge (s)) -> Terminator2/1
  Subsystem/5(SOC) -> Terminator3/1  "SOC"
  Sub3_In_Power_Consumed_by_Sub2 (kW)/1 -> [sub2]
  [sub2] -> Subsystem2/1(Sub3_Power_Consumed_by_Sub2 (kW))
  Sub3_In_Power_Consumed_by_Sub5 (kW)/1 -> [sub5]
  [sub5] -> Subsystem2/2(Sub3_Power_Consumed_by_Sub5 (kW))
  Sub3_In_Power_Consumed_by_Sub8 (kW)/1 -> [sub8]
  [sub8] -> Subsystem2/3(Sub3_Power_Consumed_by_Sub8 (kW))
  solar_power (kW)/1 -> [s_pow]
  nuclear_power (kW)/1 -> [n_pow]
  sub3_voltage/1 -> [V]
  Subsystem2/1(Sub3_Power_Concumption (kW)) -> [pow_consump]
  Subsystem/3(battery_power (kWh)) -> Product/1, [battery]
  Product/1 -> Sum/3
  [V] -> Product/2
  Sum/1 -> [pow_avail]

== system MCVT_Integration/Sys3 - Power System/Power Generation Subsystem1/Solar Power Generation ==
  Sub3_In_Solar_Irradiance : Inport
  Sub3_In_Dust : Inport  Port=2
  Sub3_In_intervension : Inport  Port=3
  Constant : Constant  Value=0
  Constant1 : Constant  Value=space_temperature
  Constant2 : Constant
  Constant3 : Constant  Value=space_temperature
  Constant4 : Constant  Value=ground_temperature
  Gain1 : Gain  Gain=1-(1-nuclear_power_toggle)*(1-solar_power_toggle)*(1-battery_power_toggle)
  MATLAB Function : MATLAB Function  TreatAsAtomicUnit=on
  MATLAB Function1 : MATLAB Function  TreatAsAtomicUnit=on
  MATLAB System : MATLABSystem  System=Solar_simu_FDD  timestep=[dt_3]  K=solar_FDD_K  noise=solar_FDD_noise  SimulateUsing=Code generation
      mask Type: Solar_simu_FDD
      mask Display:
      | disp('Solar_simu_FDD');
      | port_label('input',1,'xtrue');
      | port_label('input',2,'EnableReset');
      | port_label('input',3,'P_supply');
      | port_label('output',1,'xe');
      | port_label('output',2,'P_request');
  MATLAB System1 : MATLABSystem  System=SolarPV  PVcapacity=solar_PV_capacity  Tmodule_ini=solar_Tmodule_ini  P_ini=solar_P_ini  DRatio_ini=solar_DRatio_ini  Module_number=solar_module_num  timestep=dt_3  sigma=5.6697e-8  SimulateUsing=Code generation
      mask Type: SolarPV
      mask Display:
      | disp('SolarPV');
      | port_label('input',1,'G_in');
      | port_label('input',2,'dustrate');
      | port_label('input',3,'cleanrate');
      | port_label('input',4,'Tamb');
      | port_label('input',5,'Tsky');
      | port_label('input',6,'Tground');
      | port_label('output',1,'Pout');
      | port_label('output',2,'DRout');
  Power Switch : Constant  Value=solar_power
  Selector : Selector  InputPortWidth=4  IndexOptions=Index vector (dialog)  Indices=idx_repair_action_rate  OutputSizes=1
  Selector1 : Selector  InputPortWidth=4  IndexOptions=Index vector (dialog)  Indices=idx_Activity_Completion_Flag  OutputSizes=1
  Switch : Switch  Criteria=u2 ~= 0
  Terminator : Terminator
  Solar_Power_Output : Outport
  Sys3_solarPV_FDD : Outport  Port=2
  Sys3_solarPV_FDD_simu : Outport  Port=3
  Sub3_solarPV_DRout : Outport  Port=4
  wires:
  Sub3_In_Solar_Irradiance/1 -> MATLAB System1/1
  MATLAB System1/2 -> MATLAB Function1/1(u), Sub3_solarPV_DRout/1, MATLAB Function/1(Dratio)
  MATLAB Function/1(DustAccu) -> Gain1/1
  Sub3_In_intervension/1 -> Selector1/1, Selector/1
  Selector/1 -> MATLAB System1/3
  Constant/1 -> Switch/1
  MATLAB System1/1 -> Switch/3
  Power Switch/1 -> Switch/2
  Switch/1 -> Solar_Power_Output/1  "power_out_switch"
  Gain1/1 -> Sys3_solarPV_FDD/1
  Selector1/1 -> MATLAB System/2
  MATLAB Function1/1(y) -> MATLAB System/1
  MATLAB System/1 -> Sys3_solarPV_FDD_simu/1
  Constant2/1 -> MATLAB System/3
  MATLAB System/2 -> Terminator/1
  Sub3_In_Dust/1 -> MATLAB System1/2
  Constant1/1 -> MATLAB System1/4
  Constant3/1 -> MATLAB System1/5
  Constant4/1 -> MATLAB System1/6

== system MCVT_Integration/Sys5 - ECLSS/Active Thermal Control System (ATCS)/Faulty Panels ==
  note: Issues Found during Integration
      | - Repairs of the agent isn't consistent with the repair tiime per panel defined in Input file
      | - `dt` definitions within the block is causing issues when changed to `dt_5`
      | - Sample Time Inconsistency: Repair_ECLSS_{dust/paint} signals missnig values when entering this block
  note: Inputs
  note: Outputs
  Sys5_in_Dust deposition rate (g/cm^2) : Inport
  "Dust repairing agent (1: active, 0: inactive)" : Inport  Port=2
  "Paint repairing agent (1: active, 0: inactive)" : Inport  Port=3
  Impact : Inport  Port=4
  Repair_ECLSS_Dust : Inport  Port=5
  Repair_ECLSS_Paint : Inport  Port=6
  Bus Creator : BusCreator  Inputs=2
  Compare To Zero : simulink/Logic and Bit Operations/Compare To Zero  relop=>  OutDataTypeStr=uint8
  Compare To Zero1 : simulink/Logic and Bit Operations/Compare To Zero  relop=>  OutDataTypeStr=uint8
  Dust damage repair time : Constant  Value=ECLSS_dust_damage_repair_time
  Dust impact : MATLAB Function  TreatAsAtomicUnit=on
  Gain : Gain  Gain=RepairTime_ECLSS_factor
  Gain1 : Gain  Gain=RepairTime_ECLSS_factor
  Impact Numbering : Constant  Value=impact_numbering
  Impact Numbering1 : Constant  Value=impact_numbering
  MATLAB Function : MATLAB Function  TreatAsAtomicUnit=on
  MATLAB Function1 : MATLAB Function  TreatAsAtomicUnit=on
  MATLAB Function3 : MATLAB Function  TreatAsAtomicUnit=on
  MATLAB Function4 : MATLAB Function  TreatAsAtomicUnit=on
  Memory : Memory  InitialCondition=zeros(1,50)  InheritSampleTime=on
  Memory1 : Memory  InheritSampleTime=on
  Memory2 : Memory  InitialCondition=zeros(1,50)  InheritSampleTime=on
  Memory3 : Memory  InheritSampleTime=on
  Memory5 : Memory  InheritSampleTime=on
  Memory6 : Memory  InitialCondition=0.001  InheritSampleTime=on
  Number of panel subsection{,1..3} (4 alike) : Constant  Value=ECLSS_radiatorLoop_discreteNumber
  Override Damage Initiation : Switch  Criteria=u2 > Threshold
  Override Damage Initiation1 : Switch  Criteria=u2 > Threshold
  Paint daamge repair time : Constant  Value=ECLSS_paint_damage_repair_time
  Selector : Selector  InputPortWidth=4  IndexOptions=Index vector (dialog)  Indices=idx_failure_mode  OutputSizes=1
  Selector1 : Selector  InputPortWidth=4  IndexOptions=Index vector (dialog)  Indices=idx_failure_mode  OutputSizes=1
  Selector2 : Selector  InputPortWidth=4  IndexOptions=Index vector (dialog)  Indices=idx_Activity_Completion_Flag  OutputSizes=1
  Selector3 : Selector  InputPortWidth=4  IndexOptions=Index vector (dialog)  Indices=idx_Activity_Completion_Flag  OutputSizes=1
  Sim-FDD-ECLSS-Dust : Subsystem
  Sim-FDD-ECLSS-Paint : Subsystem
  Sys5_in_Dust parameters : Constant  Value=dust_parameters
  Sys5_in_healty panels : Constant  Value=panel_paint0
  Thermal paint damage : MATLAB Function  TreatAsAtomicUnit=on
  dt : Constant  Value=dt
  dt1 : Constant  Value=dt
  "Paint Health State(0: clean, 1:full damage)" : Outport
  "Dust Health State (0: clean, 1:full damage)" : Outport  Port=2
  faulty panels : Outport  Port=3  VectorParamsAs1DForOutWhenUnconnected=off
  Sys5_Out_Damage_Information : Outport  Port=4
  wires:
  Dust impact/1(dusty_panels) -> faulty panels/1
  Sys5_in_healty panels/1 -> Thermal paint damage/2(panel_paint0)
  Sys5_in_Dust parameters/1 -> Dust impact/3(dust_parameters)
  Override Damage Initiation/1 -> Memory/1
  MATLAB Function/3(timer) -> Memory1/1
  dt/1 -> MATLAB Function/4(dt)
  Memory/1 -> MATLAB Function/1(L_paint_k)
  Memory1/1 -> MATLAB Function/6(timer_k)
  Number of panel subsection/1 -> MATLAB Function/5(N)
  MATLAB Function/2(health) -> "Paint Health State(0: clean, 1:full damage)"/1
  MATLAB Function1/1(L_dust) -> Override Damage Initiation1/3, Sim-FDD-ECLSS-Dust/1(xtrueHS), Dust impact/1(L_dust)
  Number of panel subsection1/1 -> MATLAB Function1/6(N)
  Override Damage Initiation1/1 -> Memory2/1
  Memory2/1 -> MATLAB Function1/1(L_dust_k)
  dt1/1 -> MATLAB Function1/5(dt)
  MATLAB Function1/3(health) -> [B]
  Memory3/1 -> MATLAB Function1/7(timer_k)
  MATLAB Function1/4(timer) -> Memory3/1
  [A] -> Override Damage Initiation1/2
  [A] -> Override Damage Initiation/2
  Number of panel subsection2/1 -> MATLAB Function3/2(N)
  MATLAB Function3/1(L_dust) -> Override Damage Initiation1/1
  Number of panel subsection3/1 -> MATLAB Function4/2(N)
  MATLAB Function4/1(L_paint) -> Override Damage Initiation/1
  Memory5/1 -> MATLAB Function4/3(impact)
  Sys5_in_Dust deposition rate (g/cm^2)/1 -> MATLAB Function1/3(md)
  MATLAB Function1/5(dust_memory) -> Memory6/1
  Memory6/1 -> MATLAB Function1/8(dust_memory_k)
  Dust damage repair time/1 -> Gain/1
  Paint daamge repair time/1 -> Gain1/1
  Impact Numbering/1 -> MATLAB Function3/1(impact_numbering)
  Impact Numbering1/1 -> MATLAB Function4/1(impact_numbering)
  Impact/1 -> [A]
  [A] -> MATLAB Function3/3(impact)
  [A] -> Memory5/1
  [B] -> "Dust Health State (0: clean, 1:full damage)"/1
  Repair_ECLSS_Dust/1 -> [Repair_ECLSS_Dust]
  Repair_ECLSS_Paint/1 -> [Repair_ECLSS_Paint]
  [Repair_ECLSS_Dust] -> Selector/1
  Compare To Zero/1 -> MATLAB Function1/2(agent)
  Selector/1 -> Compare To Zero/1
  [Repair_ECLSS_Paint] -> Selector1/1
  Selector1/1 -> Compare To Zero1/1
  Compare To Zero1/1 -> MATLAB Function/2(agent)
  [Repair_ECLSS_Dust] -> Selector2/1
  Selector2/1 -> Sim-FDD-ECLSS-Dust/2(EnableResetFDD_Dust)
  [Repair_ECLSS_Paint] -> Selector3/1
  Selector3/1 -> Sim-FDD-ECLSS-Paint/2(EnableResetFDD_Paint)
  Sim-FDD-ECLSS-Dust/1(FDD_ECLSS_dust_xe) -> Bus Creator/1  "FDD_ECLSS_Dust_xe"
  Sim-FDD-ECLSS-Paint/1(FDD_ECLSS_paint_xe) -> Bus Creator/2  "FDD_ECLSS_Paint_xe"
  Bus Creator/1 -> Sys5_Out_Damage_Information/1
  MATLAB Function1/2(offset_md) -> Dust impact/2(md)
  Thermal paint damage/1(panel_paint) -> Dust impact/4(panel_paint)
  MATLAB Function/1(L_paint) -> Override Damage Initiation/3, Sim-FDD-ECLSS-Paint/1(xtrueHS), Thermal paint damage/1(L_paint)
  Gain/1 -> MATLAB Function1/4(Time)
  Gain1/1 -> MATLAB Function/3(Time)
